simulink model "TosAeb"  (R2021a)
settings: Solver=FixedStepDiscrete  StartTime=0.0  StopTime=inf  FixedStep=0.01  RelTol=1e-3
undefined symbols (not defined in the file or in its folder): ACCModSts, AccSts1, AEB_ObjectMovingOrientation, AEB_ObjectMovingState, CN_BTCActive, CN_CurrentGearR, CN_CurrentGearR_DHT, CN_PTCActive, CN_VDCActive, CN_VSC_EspOff, CN_VSC_FCW_AEB_Error, CN_VSC_FCW_AEB_NoDispay, CN_VSC_FCW_AEB_PerfmDgdtn, CN_VSC_FCW_AEB_TempUnAvlbl, CN_VSC_VehStandstill, CNaccelAvoidMax, CNaccelAvoidMin, CNbrakePedalPress, CNcomfortStrLeftAccelMax, CNcomfortStrLeftAccelMin, CNcomfortStrRightAccelMax, CNcomfortStrRightAccelMin, CNDrvDoorOpen, CNDrvModeEco, CNDrvModeNorm, CNDrvModeOther, CNDrvModeSport, CNDrvSBRreminder, CNNull, CNobjInvalidID, CNPassDoorOpen, CNPassSBRreminder, CNPEBSAccelNegInf, CNPEBSAccelNull, CNPEBSTimeInf, CNPEBSTimeNull, CNSTOPMAX, CNSTOPNULL, CNsubjWidth, CNSwtOFF, CNSwtON, CNTTCMAX, CNTTCNULL, CNVehConfigAEBCycNone, CNVehConfigAEBNone, CNVehConfigAEBPedNone, CNVehConfigFCWNone, CNVehConfigJANone, CollisionSeverity, FALSE, FCWSnvty, JACycObjCalcInfoDefaultParams, JAPedObjCalcInfoDefaultParams, JAVehObjCalcInfoDefaultParams, latCycObjCalcInfoDefaultParams, latPedObjCalcInfoDefaultParams, longObjCalcInfoDefaultParams, ModSts, movingStatus, ObjectType ... (+1112)
libraries: simulink x3031, AEBSFunctionLib x50, Ta_Library x33, AEBSStateMachineLib x23, Mylibrary x12, simulink_extras x7, ObjSelect_lib x3, dspstat3 x2
(part 56 of 102)

== system TosAeb/FcwAebJa_Step_sys/ALG_AEBSystem/ALG_AEBController/AEB_JaObjCalcApp/JaCycTgtInfoCalc/JaCycCollisonAssessInfo/JaCycCollisonAssessInfoCalc/VelAbsTgtCorrCalc/RadicalRelevantCalc ==
  dy : Inport  OutDataTypeStr=single
  dxFrontBumper : Inport  Port=2  OutDataTypeStr=single
  subjYawRate : Inport  Port=3  OutDataTypeStr=single
  Bus Creator : BusCreator  Inputs=2
  Math Function : Math  Operator=hypot  SignedPower=on  SaturateOnIntegerOverflow=on
  Product1 : Product  OutDataTypeStr=single
  Sincos : Trigonometry  Operator=sincos
  Trigonometric Function : Trigonometry  Operator=atan2
  radialVelRel : Outport
  sincosCoef : Outport  Port=2
  wires:
  subjYawRate/1 -> Product1/1
  Math Function/1 -> Product1/2  "dvRadial"
  Product1/1 -> radialVelRel/1  "radialVelRel"
  dy/1 -> Math Function/2, Trigonometric Function/1
  dxFrontBumper/1 -> Trigonometric Function/2, Math Function/1
  Trigonometric Function/1 -> Sincos/1
  Sincos/2 -> Bus Creator/2  "cosCoef"
  Bus Creator/1 -> sincosCoef/1  "sincosCoef"
  Sincos/1 -> Bus Creator/1  "sinCoef"

== system TosAeb/FcwAebJa_Step_sys/ALG_AEBSystem/ALG_AEBController/AEB_JaObjCalcApp/JaCycTgtInfoCalc/JaCycCollisonAssessInfo/JaCycCollisonAssessInfoCalc/VelAbsTgtCorrCalc/RadicalToXYTrans ==
  velRadicalXY : Inport
  sincosCoef : Inport  Port=2
  subjVel : Inport  Port=3  OutDataTypeStr=single
  Add3 : Sum  Inputs=++-  OutDataTypeStr=single
  Add4 : Sum  OutDataTypeStr=single
  Bus Creator4 : BusCreator  Inputs=2
  Bus Selector10 : BusSelector  OutputSignals=velRadicalXY.velRadicalY
  Bus Selector11 : BusSelector  OutputSignals=sincosCoef.sinCoef
  Bus Selector12 : BusSelector  OutputSignals=velRadicalXY.velRadicalX
  Bus Selector13 : BusSelector  OutputSignals=sincosCoef.sinCoef
  Bus Selector14 : BusSelector  OutputSignals=velRadicalXY.velRadicalY
  Bus Selector15 : BusSelector  OutputSignals=sincosCoef.cosCoef
  Bus Selector8 : BusSelector  OutputSignals=velRadicalXY.velRadicalX
  Bus Selector9 : BusSelector  OutputSignals=sincosCoef.cosCoef
  Product{7..10} (4 alike) : Product  OutDataTypeStr=single
  vxAbsTargetCorr : Outport
  vyAbsTargetCorr : Outport  Port=2
  wires:
  Product8/1 -> Add3/3
  Bus Selector13/1 -> Product9/2  "<sinCoef>"
  Add3/1 -> vxAbsTargetCorr/1  "vxAbsTargetCorr"
  Product7/1 -> Add3/2
  Bus Selector14/1 -> Product10/1  "<velRadicalY>"
  Bus Selector11/1 -> Product8/2  "<sinCoef>"
  Bus Selector10/1 -> Product8/1  "<velRadicalY>"
  subjVel/1 -> Add3/1
  Add4/1 -> vyAbsTargetCorr/1  "vyAbsTargetCorr"
  Bus Creator4/1 -> Bus Selector15/1, Bus Selector14/1, Bus Selector13/1, Bus Selector12/1, Bus Selector11/1, Bus Selector10/1, Bus Selector9/1, Bus Selector8/1
  Bus Selector9/1 -> Product7/2  "<cosCoef>"
  Product10/1 -> Add4/2
  Bus Selector15/1 -> Product10/2  "<cosCoef>"
  Bus Selector8/1 -> Product7/1  "<velRadicalX>"
  Product9/1 -> Add4/1
  Bus Selector12/1 -> Product9/1  "<velRadicalX>"
  velRadicalXY/1 -> Bus Creator4/1
  sincosCoef/1 -> Bus Creator4/2

== system TosAeb/FcwAebJa_Step_sys/ALG_AEBSystem/ALG_AEBController/AEB_JaObjCalcApp/JaCycTgtInfoCalc/JaCycCollisonAssessInfo/JaCycCollisonAssessInfoCalc/VelAbsTgtCorrCalc/XYToRadicalTrans ==
  LongLatVelRel : Inport
  radialVelRel : Inport  Port=2  OutDataTypeStr=single
  sincosCoef : Inport  Port=3
  Add : Sum  OutDataTypeStr=single
  Add1 : Sum  OutDataTypeStr=single
  Add2 : Sum  Inputs=+-  OutDataTypeStr=single
  Bus Creator2 : BusCreator  Inputs=2
  Bus Creator3 : BusCreator  Inputs=2
  Bus Selector : BusSelector  OutputSignals=LongLatVelRel.vx
  Bus Selector1 : BusSelector  OutputSignals=sincosCoef.cosCoef
  Bus Selector2 : BusSelector  OutputSignals=LongLatVelRel.vy
  Bus Selector3 : BusSelector  OutputSignals=sincosCoef.sinCoef
  Bus Selector4 : BusSelector  OutputSignals=LongLatVelRel.vy
  Bus Selector5 : BusSelector  OutputSignals=sincosCoef.cosCoef
  Bus Selector6 : BusSelector  OutputSignals=LongLatVelRel.vx
  Bus Selector7 : BusSelector  OutputSignals=sincosCoef.sinCoef
  Product{2,4..6} (4 alike) : Product  OutDataTypeStr=single
  velRadicalXY : Outport
  wires:
  Product6/1 -> Add2/2
  Bus Creator3/1 -> velRadicalXY/1  "velRadicalXY"
  Product5/1 -> Add2/1
  Bus Selector2/1 -> Product4/1  "<vy>"
  Product4/1 -> Add/2
  Product2/1 -> Add/1
  Bus Creator2/1 -> Bus Selector7/1, Bus Selector6/1, Bus Selector5/1, Bus Selector4/1, Bus Selector3/1, Bus Selector2/1, Bus Selector1/1, Bus Selector/1
  Bus Selector5/1 -> Product5/2  "<cosCoef>"
  Add1/1 -> Bus Creator3/2  "velRadicalY"
  Bus Selector6/1 -> Product6/1  "<vx>"
  Add/1 -> Bus Creator3/1  "velRadicalX"
  Bus Selector4/1 -> Product5/1  "<vy>"
  Bus Selector1/1 -> Product2/2  "<cosCoef>"
  radialVelRel/1 -> Add1/2
  Bus Selector3/1 -> Product4/2  "<sinCoef>"
  Bus Selector/1 -> Product2/1  "<vx>"
  Bus Selector7/1 -> Product6/2  "<sinCoef>"
  sincosCoef/1 -> Bus Creator2/2
  LongLatVelRel/1 -> Bus Creator2/1
  Add2/1 -> Add1/1

== system TosAeb/FcwAebJa_Step_sys/ALG_AEBSystem/ALG_AEBController/AEB_JaObjCalcApp/JaCycTgtInfoCalc/JaCycObjCalcInfoCalc/JaCycObjInfoCalc/ObjCrossFlgCal/CollisionProbCalc ==
  tPredTime : Inport  OutDataTypeStr=single
  subjVel : Inport  Port=2  OutDataTypeStr=single
  subjLongAccel : Inport  Port=3  OutDataTypeStr=single
  subjLatAccel : Inport  Port=4  OutDataTypeStr=single
  subjYawRate : Inport  Port=5  OutDataTypeStr=single
  ObjectTypeCameraConfirmed : Inport  Port=6
  ObjectLength : Inport  Port=7  OutDataTypeStr=single
  ObjectWidth : Inport  Port=8  OutDataTypeStr=single
  ObjectMovingOrientation : Inport  Port=9
  dx : Inport  Port=10  OutDataTypeStr=single
  dy : Inport  Port=11  OutDataTypeStr=single
  ObjectID : Inport  Port=12
  vyRelCorr : Inport  Port=13  OutDataTypeStr=single
  vxAbsObj : Inport  Port=14  OutDataTypeStr=single
  ObjectYawAngleCorr : Inport  Port=15  OutDataTypeStr=single
  function : TriggerPort  TriggerType=function-call  VariantControl=Variant  PortDimensions=1
  If : If  IfExpression=u1
  If Action Subsystem : Subsystem  TreatAsAtomicUnit=on
  Merge : Merge
  ObjIsInvalidCalc : Subsystem
  Subsystem : Subsystem
  ObjColliFlg : Outport
  wires:
  tPredTime/1 -> Subsystem/1(tPredTime)
  subjVel/1 -> Subsystem/2(subjVel)
  subjLongAccel/1 -> Subsystem/3(subjLongAccel)
  subjLatAccel/1 -> Subsystem/4(subjLatAccel)
  subjYawRate/1 -> Subsystem/5(subjYawRate)
  ObjectYawAngleCorr/1 -> Subsystem/6(ObjectYawAngleCorr)
  ObjectLength/1 -> Subsystem/8(ObjectLength)
  ObjectWidth/1 -> Subsystem/9(ObjectWidth)
  dx/1 -> Subsystem/11(dx)
  vxAbsObj/1 -> Subsystem/14(vxAbsObj)
  If/1 -> If Action Subsystem/Action
  If Action Subsystem/1(ObjColliFlg) -> Merge/1
  If/2 -> Subsystem/Action
  Merge/1 -> ObjColliFlg/1
  Subsystem/1(ObjColliFlg) -> Merge/2
  dy/1 -> Subsystem/12(dy)
  ObjectID/1 -> ObjIsInvalidCalc/1(ObjectID)
  vyRelCorr/1 -> Subsystem/13(vyRelCorr)
  ObjIsInvalidCalc/1(ObjIsInvalid) -> If/1
  ObjectMovingOrientation/1 -> Subsystem/10(ObjectMovingOrientation)
  ObjectTypeCameraConfirmed/1 -> Subsystem/7(ObjectTypeCameraConfirmed)

== system TosAeb/FcwAebJa_Step_sys/ALG_AEBSystem/ALG_AEBController/AEB_JaObjCalcApp/JaCycTgtInfoCalc/JaCycObjCalcInfoCalc/JaCycObjInfoCalc/SensorTgtInfo2AbsTgtInfoTrans1/ObjFusQualityCal/SensorDxThres ==
  subjVel : Inport
  f : TriggerPort  TriggerType=function-call  VariantControl=(inherit)
      settings: CopyFcn=Stateflow.SLINSF.SimulinkMan.removeBlockPreDeleteFcn  PreDeleteFcn=Stateflow.SLINSF.SimulinkMan.unsafePredeleteFcn
  deltaDxThresCalc : Lookup_n-D  NumberOfTableDimensions=1  BreakpointsForDimension1=P_AEB_JaCycAbs_SensorDeltDxThresX  Table=P_AEB_JaCycAbs_SensorDeltDxThresY  ExtrapMethod=Clip  InternalRulePriority=Speed  RndMeth=Simplest  InputSameDT=on
  deltaDxThres : Outport  OutDataTypeStr=single
  wires:
  subjVel/1 -> deltaDxThresCalc/1
  deltaDxThresCalc/1 -> deltaDxThres/1

== system TosAeb/FcwAebJa_Step_sys/ALG_AEBSystem/ALG_AEBController/AEB_LatObjInfoCalcApp/LatCycObjCalc/LatCycCollisonAssessInfoCalc/LatCycCollisonAssessInfoCalc/DistBrkCondCalc/CycDistBrakeFullCalc ==
  subjLongAccel : Inport  OutDataTypeStr=single
  subjVel : Inport  Port=2
  BrkSys : Subsystem
  Bus Creator : BusCreator  Inputs=6
  Bus Selector : BusSelector  OutputSignals=subjVel,tDelay,subjLongAccelFilt
  Bus Selector1 : BusSelector  OutputSignals=subjVel,Kjerk,subjLongAccelFilt,accelMax
  Bus Selector2 : BusSelector  OutputSignals=tDelay,velDelta,subjVel,subjLongAccelFilt,Kjerk,accelMax
  DistPhase1Calc : Subsystem
  DistPhase2Calc : Subsystem
  DistPhase3Calc : Subsystem
  distBrakeCalc : Subsystem
  distBrake : Outport
  wires:
  DistPhase2Calc/2(egoStopFlg) -> DistPhase3Calc/1(egoStopFlg)
  DistPhase2Calc/3(tJerkWork) -> DistPhase3Calc/2(tJerkWork)
  DistPhase2Calc/4(tPhase2) -> DistPhase3Calc/3(tPhase2)
  DistPhase3Calc/1(distPhase3) -> distBrakeCalc/3(distPhase3)
  DistPhase1Calc/1(distPhase1) -> distBrakeCalc/1(distPhase1)
  DistPhase2Calc/1(distPhase2) -> distBrakeCalc/2(distPhase2)
  distBrakeCalc/1(distBrake) -> distBrake/1
  subjVel/1 -> Bus Creator/1, BrkSys/1(subjVel)  "subjVel"
  BrkSys/3(velDelta) -> Bus Creator/4  "velDelta"
  BrkSys/4(accelMax) -> Bus Creator/5  "accelMax"
  BrkSys/2(Kjerk) -> Bus Creator/3  "Kjerk"
  BrkSys/1(tDelay) -> Bus Creator/2  "tDelay"
  BrkSys/5(subjLongAccelFilt) -> Bus Creator/6  "subjLongAccelFilt"
  Bus Creator/1 -> Bus Selector2/1, Bus Selector1/1, Bus Selector/1
  Bus Selector/3 -> DistPhase1Calc/3(subjLongAccelFilt)  "<subjLongAccelFilt>"
  Bus Selector/2 -> DistPhase1Calc/2(tPhase1)  "<tDelay>"
  Bus Selector/1 -> DistPhase1Calc/1(subjVel)  "<subjVel>"
  Bus Selector1/3 -> DistPhase2Calc/3(subjLongAccelFilt)  "<subjLongAccelFilt>"
  Bus Selector1/2 -> DistPhase2Calc/2(Kjerk)  "<Kjerk>"
  Bus Selector1/1 -> DistPhase2Calc/1(subjVel)  "<subjVel>"
  Bus Selector1/4 -> DistPhase2Calc/4(accelMax)  "<accelMax>"
  Bus Selector2/1 -> DistPhase3Calc/4(tPhase1)  "<tDelay>"
  Bus Selector2/2 -> DistPhase3Calc/5(velDelta)  "<velDelta>"
  Bus Selector2/3 -> DistPhase3Calc/6(subjVel)  "<subjVel>"
  Bus Selector2/4 -> DistPhase3Calc/7(subjLongAccelFilt)  "<subjLongAccelFilt>"
  Bus Selector2/5 -> DistPhase3Calc/8(Kjerk)  "<Kjerk>"
  Bus Selector2/6 -> DistPhase3Calc/9(accelMax)  "<accelMax>"
  subjLongAccel/1 -> BrkSys/2(subjLongAccel)

== system TosAeb/FcwAebJa_Step_sys/ALG_AEBSystem/ALG_AEBController/AEB_LatObjInfoCalcApp/LatCycObjCalc/LatCycCollisonAssessInfoCalc/LatCycCollisonAssessInfoCalc/DistBrkCondCalc/CycDistBrakePartCalc ==
  subjLongAccel : Inport  OutDataTypeStr=single
  subjVel : Inport  Port=2
  BrkSys : Subsystem
  Bus Creator : BusCreator  Inputs=6
  Bus Selector : BusSelector  OutputSignals=subjVel,tDelay,subjLongAccelFilt
  Bus Selector1 : BusSelector  OutputSignals=subjVel,Kjerk,subjLongAccelFilt,accelMax
  Bus Selector2 : BusSelector  OutputSignals=tDelay,velDelta,subjVel,subjLongAccelFilt,Kjerk,accelMax
  DistPhase1Calc : Subsystem
  DistPhase2Calc : Subsystem
  DistPhase3Calc : Subsystem
  distBrakeCalc : Subsystem
  distBrake : Outport
  wires:
  subjLongAccel/1 -> BrkSys/2(subjLongAccel)
  Bus Selector2/6 -> DistPhase3Calc/9(accelMax)  "<accelMax>"
  Bus Selector2/5 -> DistPhase3Calc/8(Kjerk)  "<Kjerk>"
  Bus Selector2/4 -> DistPhase3Calc/7(subjLongAccelFilt)  "<subjLongAccelFilt>"
  Bus Selector2/3 -> DistPhase3Calc/6(subjVel)  "<subjVel>"
  Bus Selector2/2 -> DistPhase3Calc/5(velDelta)  "<velDelta>"
  Bus Selector2/1 -> DistPhase3Calc/4(tPhase1)  "<tDelay>"
  Bus Selector1/4 -> DistPhase2Calc/4(accelMax)  "<accelMax>"
  Bus Selector1/1 -> DistPhase2Calc/1(subjVel)  "<subjVel>"
  Bus Selector1/2 -> DistPhase2Calc/2(Kjerk)  "<Kjerk>"
  Bus Selector1/3 -> DistPhase2Calc/3(subjLongAccelFilt)  "<subjLongAccelFilt>"
  Bus Selector/1 -> DistPhase1Calc/1(subjVel)  "<subjVel>"
  Bus Selector/2 -> DistPhase1Calc/2(tPhase1)  "<tDelay>"
  Bus Selector/3 -> DistPhase1Calc/3(subjLongAccelFilt)  "<subjLongAccelFilt>"
  Bus Creator/1 -> Bus Selector/1, Bus Selector1/1, Bus Selector2/1
  BrkSys/5(subjLongAccelFilt) -> Bus Creator/6  "subjLongAccelFilt"
  BrkSys/1(tDelay) -> Bus Creator/2  "tDelay"
  BrkSys/2(Kjerk) -> Bus Creator/3  "Kjerk"
  BrkSys/4(accelMax) -> Bus Creator/5  "accelMax"
  BrkSys/3(velDelta) -> Bus Creator/4  "velDelta"
  subjVel/1 -> BrkSys/1(subjVel), Bus Creator/1  "subjVel"
  distBrakeCalc/1(distBrake) -> distBrake/1
  DistPhase2Calc/1(distPhase2) -> distBrakeCalc/2(distPhase2)
  DistPhase1Calc/1(distPhase1) -> distBrakeCalc/1(distPhase1)
  DistPhase3Calc/1(distPhase3) -> distBrakeCalc/3(distPhase3)
  DistPhase2Calc/4(tPhase2) -> DistPhase3Calc/3(tPhase2)
  DistPhase2Calc/3(tJerkWork) -> DistPhase3Calc/2(tJerkWork)
  DistPhase2Calc/2(egoStopFlg) -> DistPhase3Calc/1(egoStopFlg)

== system TosAeb/FcwAebJa_Step_sys/ALG_AEBSystem/ALG_AEBController/AEB_LatObjInfoCalcApp/LatCycObjCalc/LatCycCollisonAssessInfoCalc/LatCycCollisonAssessInfoCalc/DistBrkCondCalc/distBraking4FullBrakeCalc1 ==
  subLongAccel : Inport  OutDataTypeStr=single
  subjVel : Inport  Port=2
  Bus Creator : BusCreator  Inputs=2
  Bus Selector : BusSelector  OutputSignals=brkSysAtribute.subjLongAccelCorr,brkSysAtribute.tdelay,subjVel
  Bus Selector1 : BusSelector  OutputSignals=brkSysAtribute.accelMax,brkSysAtribute.subjLongAccelCorr,brkSysAtribute.Kjerk,subjVel
  Bus Selector2 : BusSelector  OutputSignals=brkSysAtribute.subjLongAccelCorr,brkSysAtribute.Kjerk,brkSysAtribute.subjLongAccelCorr,brkSysAtribute.velReduceMax,brkSysAtribute.accelMax
  DistPhase1Calc : Subsystem
  DistPhase2Calc : Subsystem
  brkSysAtributeCalc : Subsystem
  distPhase3Calc : Subsystem
  distanceTriggerBrakingCalc : Subsystem
  distBraking4FullBrake : Outport
  wires:
  Bus Selector2/1 -> distPhase3Calc/3(subjLongAccelCorr)  "<subjLongAccelCorr>"
  Bus Selector2/2 -> distPhase3Calc/4(Kjerk)  "<Kjerk>"
  Bus Selector2/5 -> distPhase3Calc/7(accelMax)  "<accelMax>"
  Bus Selector2/3 -> distPhase3Calc/5(accelWorkPhase1)  "<subjLongAccelCorr>"
  Bus Selector2/4 -> distPhase3Calc/6(velMaxReduce)  "<velReduceMax>"
  [timeWorkPhase1] -> distPhase3Calc/8(tWorkPhase1)
  DistPhase1Calc/2(timeWorkPhase1) -> [timeWorkPhase1]
  DistPhase2Calc/2(Phase2EgoStopFlg) -> distPhase3Calc/1(Phase2EgoStopFlg)
  Bus Selector1/4 -> DistPhase2Calc/4(subjVel)  "<subjVel>"
  DistPhase2Calc/3(tWorkPhase2) -> distPhase3Calc/2(tWorkPhase2)
  brkSysAtributeCalc/1(brkSysAtribute) -> Bus Creator/1  "brkSysAtribute"
  DistPhase1Calc/1(distPhase1) -> distanceTriggerBrakingCalc/1(distPhase1)
  subjVel/1 -> brkSysAtributeCalc/1(subjVel), Bus Creator/2  "subjVel"
  distPhase3Calc/1(distPhase3) -> distanceTriggerBrakingCalc/3(distPhase3)
  subLongAccel/1 -> brkSysAtributeCalc/2(subLongAccel)
  Bus Selector1/2 -> DistPhase2Calc/2(accelWorkPhase1)  "<subjLongAccelCorr>"
  Bus Selector1/1 -> DistPhase2Calc/1(accelMax)  "<accelMax>"
  DistPhase2Calc/1(distPhase2) -> distanceTriggerBrakingCalc/2(distPhase2)
  Bus Selector/1 -> DistPhase1Calc/1(subjlongAccelCorr)  "<subjLongAccelCorr>"
  Bus Selector1/3 -> DistPhase2Calc/3(Kjerk)  "<Kjerk>"
  Bus Selector/3 -> DistPhase1Calc/3(subjVel)  "<subjVel>"
  Bus Selector/2 -> DistPhase1Calc/2(tdelay)  "<tdelay>"
  Bus Creator/1 -> Bus Selector/1, Bus Selector2/1, Bus Selector1/1
  distanceTriggerBrakingCalc/1(DistBraking4FullBrake) -> distBraking4FullBrake/1

== system TosAeb/FcwAebJa_Step_sys/ALG_AEBSystem/ALG_AEBController/AEB_LatObjInfoCalcApp/LatCycObjCalc/LatCycCollisonAssessInfoCalc/LatCycCollisonAssessInfoCalc/DistBrkCondCalc/distBraking4PartBrakeCalc1 ==
  subLongAccel : Inport  OutDataTypeStr=single
  subjVel : Inport  Port=2
  Bus Creator : BusCreator  Inputs=2
  Bus Selector : BusSelector  OutputSignals=brkSysAtribute.subjLongAccelCorr,brkSysAtribute.tdelay,subjVel
  Bus Selector1 : BusSelector  OutputSignals=brkSysAtribute.accelMax,brkSysAtribute.subjLongAccelCorr,brkSysAtribute.Kjerk,subjVel
  Bus Selector2 : BusSelector  OutputSignals=brkSysAtribute.subjLongAccelCorr,brkSysAtribute.Kjerk,brkSysAtribute.subjLongAccelCorr,brkSysAtribute.velReduceMax,brkSysAtribute.accelMax
  DistPhase1Calc : Subsystem
  DistPhase2Calc : Subsystem
  brkSysAtributeCalc : Subsystem
  distPhase3Calc : Subsystem
  distanceTriggerBrakingCalc : Subsystem
  distBraking4PartBrake : Outport
  wires:
  distanceTriggerBrakingCalc/1(distBraking4PartBrake) -> distBraking4PartBrake/1
  Bus Creator/1 -> Bus Selector1/1, Bus Selector2/1, Bus Selector/1
  Bus Selector/2 -> DistPhase1Calc/2(tdelay)  "<tdelay>"
  Bus Selector/3 -> DistPhase1Calc/3(subjVel)  "<subjVel>"
  Bus Selector1/3 -> DistPhase2Calc/3(Kjerk)  "<Kjerk>"
  Bus Selector/1 -> DistPhase1Calc/1(subjlongAccelCorr)  "<subjLongAccelCorr>"
  DistPhase2Calc/1(distPhase2) -> distanceTriggerBrakingCalc/2(distPhase2)
  Bus Selector1/1 -> DistPhase2Calc/1(accelMax)  "<accelMax>"
  Bus Selector1/2 -> DistPhase2Calc/2(accelWorkPhase1)  "<subjLongAccelCorr>"
  subLongAccel/1 -> brkSysAtributeCalc/2(subLongAccel)
  distPhase3Calc/1(distPhase3) -> distanceTriggerBrakingCalc/3(distPhase3)
  subjVel/1 -> Bus Creator/2, brkSysAtributeCalc/1(subjVel)  "subjVel"
  DistPhase1Calc/1(distPhase1) -> distanceTriggerBrakingCalc/1(distPhase1)
  brkSysAtributeCalc/1(brkSysAtribute) -> Bus Creator/1  "brkSysAtribute"
  DistPhase2Calc/3(tWorkPhase2) -> distPhase3Calc/2(tWorkPhase2)
  Bus Selector1/4 -> DistPhase2Calc/4(subjVel)  "<subjVel>"
  DistPhase2Calc/2(Phase2EgoStopFlg) -> distPhase3Calc/1(Phase2EgoStopFlg)
  DistPhase1Calc/2(timeWorkPhase1) -> [timeWorkPhase1]
  [timeWorkPhase1] -> distPhase3Calc/8(tWorkPhase1)
  Bus Selector2/4 -> distPhase3Calc/6(velMaxReduce)  "<velReduceMax>"
  Bus Selector2/3 -> distPhase3Calc/5(accelWorkPhase1)  "<subjLongAccelCorr>"
  Bus Selector2/5 -> distPhase3Calc/7(accelMax)  "<accelMax>"
  Bus Selector2/2 -> distPhase3Calc/4(Kjerk)  "<Kjerk>"
  Bus Selector2/1 -> distPhase3Calc/3(subjLongAccelCorr)  "<subjLongAccelCorr>"

== system TosAeb/FcwAebJa_Step_sys/ALG_AEBSystem/ALG_AEBController/AEB_LatObjInfoCalcApp/LatCycObjCalc/LatCycCollisonAssessInfoCalc/LatCycCollisonAssessInfoCalc/DistBrkCondCalc/distSecurCalc ==
  subVel : Inport
  Compare To Constant : simulink/Logic and Bit Operations/Compare To Constant  relop=>  const=P_AEB_LatCycCA_DxSecureSubjVelThres  OutDataTypeStr=boolean
  If : If  IfExpression=u1
  If Action Subsystem : Subsystem  TreatAsAtomicUnit=on
  If Action Subsystem1 : Subsystem  TreatAsAtomicUnit=on
  Merge : Merge
  distSecure : Outport
  wires:
  Merge/1 -> distSecure/1
  If Action Subsystem/1(distSecure) -> Merge/2
  If Action Subsystem1/1(distSecure) -> Merge/1
  If/2 -> If Action Subsystem/Action
  If/1 -> If Action Subsystem1/Action
  Compare To Constant/1 -> If/1
  subVel/1 -> Compare To Constant/1, If Action Subsystem/1(subjVel)

== system TosAeb/FcwAebJa_Step_sys/ALG_AEBSystem/ALG_AEBController/AEB_LatObjInfoCalcApp/LatCycObjCalc/LatCycObjCalc/TgtPreSelectCalc/TgtPreSelect/Object Cond  ==
  ObjectType : Inport
  ObjectTypeCameraConfirmed : Inport  Port=2
  ObjectIsObstacleProb : Inport  Port=3
  Compare To Constant6 : simulink/Logic and Bit Operations/Compare To Constant  relop===  const=TRUE  OutDataTypeStr=boolean
  Compare To Constant7 : simulink/Logic and Bit Operations/Compare To Constant  relop===  const=ObjectType.BICYCLE  OutDataTypeStr=boolean
  Compare To Constant8 : simulink/Logic and Bit Operations/Compare To Constant  relop===  const=ObjectType.BICYCLE  OutDataTypeStr=boolean
  Compare To Constant9 : simulink/Logic and Bit Operations/Compare To Constant  relop=>  const=P_AEB_LatCycCA_PreSelectObjectIsObstacleProbThres  OutDataTypeStr=boolean
  Logical Operator : Logic  AllPortsSameDT=off  OutDataTypeStr=boolean
  Switch : Switch  Criteria=u2 ~= 0
  Out1 : Outport
  wires:
  Switch/1 -> Out1/1
  Logical Operator/1 -> Switch/3
  Compare To Constant9/1 -> Logical Operator/2
  ObjectType/1 -> Compare To Constant7/1, Compare To Constant8/1
  Compare To Constant7/1 -> Logical Operator/1
  Compare To Constant6/1 -> Switch/2
  ObjectTypeCameraConfirmed/1 -> Compare To Constant6/1
  Compare To Constant8/1 -> Switch/1
  ObjectIsObstacleProb/1 -> Compare To Constant9/1

== system TosAeb/FcwAebJa_Step_sys/ALG_AEBSystem/ALG_AEBController/AEB_LatObjInfoCalcApp/LatCycObjCalc/LatCycObjCalc/latCycObjInfoCalc/ObjColiisionRiskProbCalc/CollisionRiskProbCalc ==
  tPredTime : Inport
  ObjectTypeCameraConfirmed : Inport  Port=2
  ObjectLength : Inport  Port=3
  ObjectWidth : Inport  Port=4
  ObjectYawAngle : Inport  Port=5
  ObjectMovingOrientation : Inport  Port=6
  vx : Inport  Port=7
  vy : Inport  Port=8
  dy : Inport  Port=9
  dx : Inport  Port=10
  subjVel : Inport  Port=11
  subjYawRate : Inport  Port=12
  dxFrontBumper : Inport  Port=13
  vxAbsObj : Inport  Port=14
  function : TriggerPort  TriggerType=function-call  VariantControl=Variant  PortDimensions=1
  Bus Creator : BusCreator  Inputs=14
  Bus Selector : BusSelector  OutputSignals=tPredTime,subjVel
  Bus Selector1 : BusSelector  OutputSignals=vx,vy,dy,dxFrontBumper,subjYawRate,subjVel
  Bus Selector2 : BusSelector  OutputSignals=tPredTime,ObjectTypeCameraConfirmed,ObjectWidth,ObjectYawAngle,ObjectMovingOrientation,ObjectLength,dx,dy,vxAbsObj,vy
  CollisionRiskProbCalc : Subsystem
  Display : Display
  Display1 : Display
  Display2 : Display
  EgoReginCalc : Subsystem
  EgoReginCorrCalc : Subsystem
  MATLAB Function : MATLAB Function  Commented=on  TreatAsAtomicUnit=on
  ObjAbsInfoCalc : Subsystem
  ObjReginCorrCalc : Subsystem
  Terminator1 : Terminator
  Terminator2 : Terminator
  collisionRiskProb : Outport
  dyCollisionRiskProb : Outport  Port=2
  dxCollisionRiskProb : Outport  Port=3
  wires:
  CollisionRiskProbCalc/5(dxCollisionRiskProb) -> Display1/1, [dxCollisionRiskProb], dxCollisionRiskProb/1
  CollisionRiskProbCalc/4(dyCollisionRiskProb) -> Display/1, [dyCollisionRiskProb], dyCollisionRiskProb/1
  ObjReginCorrCalc/4(dyReginObjLLCorr) -> CollisionRiskProbCalc/8(dyReginObjLLCorr), [dyReginObjLLCorr]
  ObjReginCorrCalc/3(dxReginObjLLCorr) -> CollisionRiskProbCalc/7(dxReginObjLLCorr), [dxReginObjLLCorr]
  ObjReginCorrCalc/2(dyReginObjRUCorr) -> CollisionRiskProbCalc/6(dyReginObjRUCorr), [dyReginObjRUCorr]
  ObjReginCorrCalc/1(dxReginObjRUCorr) -> CollisionRiskProbCalc/5(dxReginObjRUCorr), [dxReginObjRUCorr]
  EgoReginCalc/4(dyReginEgoLL) -> EgoReginCorrCalc/4(dyReginEgoLL)
  EgoReginCalc/3(dxReginEgoLL) -> EgoReginCorrCalc/3(dxReginEgoLL)
  EgoReginCalc/2(dyReginEgoRU) -> EgoReginCorrCalc/2(dyReginEgoRU)
  EgoReginCalc/1(dxReginEgoRU) -> EgoReginCorrCalc/1(dxReginEgoRU)
  Bus Selector1/6 -> ObjAbsInfoCalc/6(subjVel)  "<subjVel>"
  Bus Selector1/5 -> ObjAbsInfoCalc/5(subjYawRate)  "<subjYawRate>"
  Bus Selector1/4 -> ObjAbsInfoCalc/4(dxFrontBumper)  "<dxFrontBumper>"
  Bus Selector1/3 -> ObjAbsInfoCalc/3(dy)  "<dy>"
  Bus Selector1/2 -> ObjAbsInfoCalc/2(vy)  "<vy>"
  Bus Selector1/1 -> ObjAbsInfoCalc/1(vx)  "<vx>"
  subjYawRate/1 -> Bus Creator/13  "subjYawRate"
  subjVel/1 -> Bus Creator/12  "subjVel"
  dxFrontBumper/1 -> Bus Creator/11  "dxFrontBumper"
  dy/1 -> [dy], Bus Creator/10  "dy"
  dx/1 -> [dx], Bus Creator/9  "dx"
  Bus Selector/2 -> EgoReginCalc/2(subjVel)  "<subjVel>"
  Bus Selector/1 -> EgoReginCalc/1(tPredTime)  "<tPredTime>"
  Bus Creator/1 -> Bus Selector2/1, Bus Selector1/1, Bus Selector/1
  vy/1 -> Bus Creator/8  "vy"
  vx/1 -> Bus Creator/7  "vx"
  ObjectYawAngle/1 -> Bus Creator/5  "ObjectYawAngle"
  ObjectWidth/1 -> Bus Creator/4  "ObjectWidth"
  ObjectLength/1 -> Bus Creator/3  "ObjectLength"
  ObjectTypeCameraConfirmed/1 -> Bus Creator/2  "ObjectTypeCameraConfirmed"
  tPredTime/1 -> Bus Creator/1  "tPredTime"
  ObjAbsInfoCalc/1(velAbsObjCorr) -> ObjReginCorrCalc/1(velAbsObjCorr)
  CollisionRiskProbCalc/3(collisionRiskProb) -> Display2/1, [collisionRiskProb], collisionRiskProb/1
  CollisionRiskProbCalc/2(dyPreCollison) -> Terminator2/1
  CollisionRiskProbCalc/1(dxPreCollison) -> Terminator1/1
  EgoReginCorrCalc/2(dyReginEgoRUCorr) -> [dyReginEgoRUCorr], CollisionRiskProbCalc/2(dyReginEgoRUCorr)
  EgoReginCorrCalc/3(dyReginEgoLLCorr) -> [dyReginEgoLLCorr], CollisionRiskProbCalc/3(dyReginEgoLLCorr)
  EgoReginCorrCalc/1(dxReginEgoRUCorr) -> [dxReginEgoRUCorr], CollisionRiskProbCalc/1(dxReginEgoRUCorr)
  EgoReginCorrCalc/4(dxReginEgoLLCorr) -> [dxReginEgoLLCorr], CollisionRiskProbCalc/4(dxReginEgoLLCorr)
  Bus Selector2/1 -> ObjReginCorrCalc/2(tPredTime)  "<tPredTime>"
  Bus Selector2/3 -> ObjReginCorrCalc/4(ObjectWidth)  "<ObjectWidth>"
  Bus Selector2/4 -> ObjReginCorrCalc/5(ObjectYawAngle)  "<ObjectYawAngle>"
  Bus Selector2/6 -> ObjReginCorrCalc/7(ObjectLength)  "<ObjectLength>"
  Bus Selector2/7 -> ObjReginCorrCalc/8(dx)  "<dx>"
  Bus Selector2/8 -> ObjReginCorrCalc/9(dy)  "<dy>"
  Bus Selector2/9 -> ObjReginCorrCalc/10(vxAbsObj)  "<vxAbsObj>"
  Bus Selector2/10 -> ObjReginCorrCalc/11(vy)  "<vy>"
  vxAbsObj/1 -> Bus Creator/14  "vxAbsObj"
  [dyReginObjRUCorr] -> MATLAB Function/6(dyReginObjRUCorr)
  [dyCollisionRiskProb] -> MATLAB Function/12(dyCollisionRiskProb)
  [collisionRiskProb] -> MATLAB Function/11(collisionRiskProb)
  [dxCollisionRiskProb] -> MATLAB Function/13(dxCollisionRiskProb)
  [dxReginObjLLCorr] -> MATLAB Function/7(dxReginObjLLCorr)
  [dyReginObjLLCorr] -> MATLAB Function/8(dyReginObjLLCorr)
  [dxReginEgoRUCorr] -> MATLAB Function/1(dxReginEgoRUCorr)
  [dxReginObjRUCorr] -> MATLAB Function/5(dxReginObjRUCorr)
  [dxReginEgoLLCorr] -> MATLAB Function/4(dxReginEgoLLCorr)
  [dyReginEgoLLCorr] -> MATLAB Function/3(dyReginEgoLLCorr)
  [dyReginEgoRUCorr] -> MATLAB Function/2(dyReginEgoRUCorr)
  [dx] -> MATLAB Function/9(dx)
  [dy] -> MATLAB Function/10(dy)
  ObjectMovingOrientation/1 -> Bus Creator/6
  Bus Selector2/5 -> ObjReginCorrCalc/6(ObjectMovingOrientation)  "<ObjectMovingOrientation>"
  Bus Selector2/2 -> ObjReginCorrCalc/3(ObjectTypeCameraConfirmed)  "<ObjectTypeCameraConfirmed>"

== system TosAeb/FcwAebJa_Step_sys/ALG_AEBSystem/ALG_AEBController/AEB_LatObjInfoCalcApp/LatCycObjCalc/LatCycObjCalc/latCycObjInfoCalc/ObjCrossFlgCal/CycPreCollisionCalcFunc ==
  tPredTime : Inport  OutDataTypeStr=single
  subjVel : Inport  Port=2
  subjLongAccel : Inport  Port=3
  subjLatAccel : Inport  Port=4
  subjYawRate : Inport  Port=5
  ObjectTypeCameraConfirmed : Inport  Port=6
  ObjectLength : Inport  Port=7
  ObjectWidth : Inport  Port=8
  ObjectYawAngle : Inport  Port=9
  ObjectMovingOrientation : Inport  Port=10
  dx : Inport  Port=11
  vy : Inport  Port=12
  ay : Inport  Port=13
  dy : Inport  Port=14
  vxAbsObj : Inport  Port=15
  axAbsObj : Inport  Port=16
  function : TriggerPort  TriggerType=function-call  VariantControl=Variant  PortDimensions=1
  ObjBoundingBox : Subsystem
  ObjLenthWidthCalc : Subsystem
  ObjPreReferPointCalc : Subsystem
  PreCrossFlgCalcFlow : Stateflow chart  TreatAsAtomicUnit=on
  PreRefPointEgoCalc : Subsystem
  SubjBoundingBox : Subsystem
  sincosCoefCalc : Subsystem
  sincosCoefCalc1 : Subsystem
  pedPreCollisionFlg : Outport
  wires:
  [subjLatAccel] -> ObjPreReferPointCalc/6(subjLatAccel)
  [tPredTime] -> ObjPreReferPointCalc/3(tPredTime)
  subjYawRate/1 -> PreRefPointEgoCalc/5(subjYawRate)
  subjLatAccel/1 -> PreRefPointEgoCalc/4(subjLatAccel), [subjLatAccel]
  subjVel/1 -> PreRefPointEgoCalc/1(subjVel)
  subjLongAccel/1 -> PreRefPointEgoCalc/3(subjLongAccel)
  tPredTime/1 -> PreRefPointEgoCalc/2(tPredTime), [tPredTime]
  dy/1 -> ObjPreReferPointCalc/8(dy)
  ay/1 -> ObjPreReferPointCalc/7(ay)
  vy/1 -> ObjPreReferPointCalc/5(vy)
  axAbsObj/1 -> ObjPreReferPointCalc/4(axAbsObj)
  vxAbsObj/1 -> ObjPreReferPointCalc/2(vxAbsObj)
  dx/1 -> ObjPreReferPointCalc/1(dx)
  ObjectWidth/1 -> ObjLenthWidthCalc/3(ObjectWidth)
  ObjectLength/1 -> ObjLenthWidthCalc/2(ObjectLength)
  PreCrossFlgCalcFlow/1(pedPreCollisionFlg) -> pedPreCollisionFlg/1
  ObjBoundingBox/2(objDyPre) -> PreCrossFlgCalcFlow/4(objDyPre)
  ObjBoundingBox/1(objDxPre) -> PreCrossFlgCalcFlow/3(objDxPre)
  SubjBoundingBox/2(subjDyPre) -> PreCrossFlgCalcFlow/2(subjDyPre)
  SubjBoundingBox/1(subjDxPre) -> PreCrossFlgCalcFlow/1(subjDxPre)
  sincosCoefCalc1/1(sincosCoef) -> ObjBoundingBox/5(sincosCoef)
  ObjPreReferPointCalc/2(dyPreRefPointObj) -> ObjBoundingBox/4(dyPreRefPointObj)
  ObjPreReferPointCalc/1(dxPreRefPointObj) -> ObjBoundingBox/3(dxPreRefPointObj)
  ObjLenthWidthCalc/1(ObjLengthCorr) -> ObjBoundingBox/1(ObjLengthCorr)
  ObjLenthWidthCalc/2(ObjWidthCorr) -> ObjBoundingBox/2(ObjWidthCorr)
  ObjPreReferPointCalc/3(YawAnglePreObj) -> sincosCoefCalc1/1(angle)
  PreRefPointEgoCalc/1(" dxPreRefPoint") -> SubjBoundingBox/1(dxPreRefPoint)
  sincosCoefCalc/1(sincosCoef) -> SubjBoundingBox/3(sincosCoef)
  PreRefPointEgoCalc/2(" dyPreRefPoint") -> SubjBoundingBox/2(dyPreRefPoint)
  PreRefPointEgoCalc/3(YawAnglePre) -> sincosCoefCalc/1(angle)
  ObjectMovingOrientation/1 -> ObjLenthWidthCalc/5(ObjectMovingOrientation)
  ObjectTypeCameraConfirmed/1 -> ObjLenthWidthCalc/1(ObjectTypeCameraConfirmed)
  ObjectYawAngle/1 -> ObjPreReferPointCalc/9(ObjectYawAngle), ObjLenthWidthCalc/4(ObjectYawAngle)

== system TosAeb/FcwAebJa_Step_sys/ALG_AEBSystem/ALG_AEBController/AEB_LatObjInfoCalcApp/LatCycObjCalc/LatCycObjCalc/latCycObjInfoCalc/SensorTgtInfo2AbsTgtInfoTrans/ObjFusQualityCal/SensorVyThres ==
  subjVel : Inport
  f : TriggerPort  TriggerType=function-call  VariantControl=(inherit)
      settings: CopyFcn=Stateflow.SLINSF.SimulinkMan.removeBlockPreDeleteFcn  PreDeleteFcn=Stateflow.SLINSF.SimulinkMan.unsafePredeleteFcn
  deltaDxThresCalc : Lookup_n-D  NumberOfTableDimensions=1  BreakpointsForDimension1=P_AEB_LatCycObjAbs_SensorDeltDxThresX  Table=P_AEB_LatCycObjAbs_SensorDeltDxThresY  ExtrapMethod=Clip  InternalRulePriority=Speed  RndMeth=Simplest  InputSameDT=on
  deltaDyThresCalc : Lookup_n-D  NumberOfTableDimensions=1  BreakpointsForDimension1=P_AEB_LatCycObjAbs_SensorDeltDyThresX  Table=P_AEB_LatCycObjAbs_SensorDeltDyThresY  ExtrapMethod=Clip  InternalRulePriority=Speed  RndMeth=Simplest  InputSameDT=on
  deltaVyThresCalc : Lookup_n-D  NumberOfTableDimensions=1  BreakpointsForDimension1=P_AEB_LatCycObjAbs_SensorDeltVyThresX  Table=P_AEB_LatCycObjAbs_SensorDeltVyThresY  ExtrapMethod=Clip  InternalRulePriority=Speed  RndMeth=Simplest  InputSameDT=on
  deltaDxThres : Outport  OutDataTypeStr=single
  deltaDyThres : Outport  Port=2  OutDataTypeStr=single
  deltaVyThres : Outport  Port=3  OutDataTypeStr=single
  wires:
  subjVel/1 -> deltaDxThresCalc/1, deltaDyThresCalc/1, deltaVyThresCalc/1
  deltaVyThresCalc/1 -> deltaVyThres/1
  deltaDxThresCalc/1 -> deltaDxThres/1
  deltaDyThresCalc/1 -> deltaDyThres/1

== system TosAeb/FcwAebJa_Step_sys/ALG_AEBSystem/ALG_AEBController/AEB_LatObjInfoCalcApp/LatPedObjCalc/LatPedCollisonAssessInfo/LatPedCollisonAssessInfo/LatPedCollisionSeverityCalc/longDistCond4CollisionSeverityHighCalc ==
  distSecure : Inport
  distBraking : Inport  Port=2  OutDataTypeStr=single
  dxFrontBumper : Inport  Port=3  OutDataTypeStr=single
  vx : Inport  Port=4
  Add : Sum  Inputs=++-  OutDataTypeStr=single
  P_LatPedCA_tSeverityHighTimeOffset : Constant  Value=P_AEB_LatPedCA_tSeverityHighTimeOffset
  Product : Product
  Relational Operator : RelationalOperator  Operator=<=  OutDataTypeStr=boolean  RndMeth=Simplest
  longDistCond4CollisionSeverityHigh : Outport
  wires:
  Add/1 -> Relational Operator/2
  vx/1 -> Product/1
  dxFrontBumper/1 -> Relational Operator/1
  Relational Operator/1 -> longDistCond4CollisionSeverityHigh/1
  distSecure/1 -> Add/1
  distBraking/1 -> Add/2
  P_LatPedCA_tSeverityHighTimeOffset/1 -> Product/2
  Product/1 -> Add/3

== system TosAeb/FcwAebJa_Step_sys/ALG_AEBSystem/ALG_AEBController/AEB_LatObjInfoCalcApp/LatPedObjCalc/LatPedCollisonAssessInfo/LatPedCollisonAssessInfo/LatPedCollisionSeverityCalc/longDistCond4CollisionSeverityLowCalc ==
  dxFrontBumper : Inport  OutDataTypeStr=single
  distSecure : Inport  Port=2
  distBraking : Inport  Port=3  OutDataTypeStr=single
  subjVel : Inport  Port=4
  Add : Sum  Inputs=+++  OutDataTypeStr=single
  P_LatPedCA_SeverityLowTimeOffset : Constant  Value=P_AEB_LatPedCA_SeverityLowTimeOffset
  Product : Product
  Relational Operator : RelationalOperator  Operator=<=  OutDataTypeStr=boolean  RndMeth=Simplest
  longDistCond4CollisionSeverityLow : Outport
  wires:
  distSecure/1 -> Add/1
  distBraking/1 -> Add/2
  Add/1 -> Relational Operator/2
  dxFrontBumper/1 -> Relational Operator/1
  Relational Operator/1 -> longDistCond4CollisionSeverityLow/1
  subjVel/1 -> Product/1
  Product/1 -> Add/3
  P_LatPedCA_SeverityLowTimeOffset/1 -> Product/2

== system TosAeb/FcwAebJa_Step_sys/ALG_AEBSystem/ALG_AEBController/AEB_LatObjInfoCalcApp/LatPedObjCalc/LatPedCollisonAssessInfo/LatPedCollisonAssessInfo/LatPedCollisionSeverityCalc/longDistCond4CollisionSeverityMediumCalc ==
  FcwHMISetting : Inport
  subjVel : Inport  Port=2
  dxFrontBumper : Inport  Port=3  OutDataTypeStr=single
  Compare To Constant : simulink/Logic and Bit Operations/Compare To Constant  relop===  const=FCWSnvty.HMISettingEarly  OutDataTypeStr=boolean
  Compare To Constant1 : simulink/Logic and Bit Operations/Compare To Constant  relop===  const=FCWSnvty.HMISettingMiddle  OutDataTypeStr=boolean
  Logical Operator : Logic  Operator=OR  AllPortsSameDT=off  OutDataTypeStr=boolean
  P_LatPedCA_SeverityMediumMaxTime : Constant  Value=P_AEB_LatPedCA_SeverityMediumMaxTime  OutDataTypeStr=single
  P_LatPedCA_SeverityMediumMaxTimeHmiLate : Constant  Value=P_AEB_LatPedCA_SeverityMediumMaxTimeHmiLate  OutDataTypeStr=single
  Product : Product  OutDataTypeStr=single
  Relational Operator : RelationalOperator  Operator=<=  OutDataTypeStr=boolean  RndMeth=Simplest
  Switch : Switch  Criteria=u2 ~= 0  OutDataTypeStr=single
  longDistCond4CollisionSeverityMedium : Outport
  wires:
  FcwHMISetting/1 -> Compare To Constant1/1, Compare To Constant/1
  Compare To Constant/1 -> Logical Operator/1
  Compare To Constant1/1 -> Logical Operator/2
  Logical Operator/1 -> Switch/2
  P_LatPedCA_SeverityMediumMaxTime/1 -> Switch/1
  P_LatPedCA_SeverityMediumMaxTimeHmiLate/1 -> Switch/3
  Switch/1 -> Product/1
  subjVel/1 -> Product/2
  Product/1 -> Relational Operator/2
  dxFrontBumper/1 -> Relational Operator/1
  Relational Operator/1 -> longDistCond4CollisionSeverityMedium/1

== system TosAeb/FcwAebJa_Step_sys/ALG_AEBSystem/ALG_AEBController/AEB_LatObjInfoCalcApp/LatPedObjCalc/LatPedCollisonAssessInfo/LatPedCollisonAssessInfo/LatPedLongDistCondCalc/DistSecureCalc ==
  subjVel : Inport
  Compare To Constant : simulink/Logic and Bit Operations/Compare To Constant  relop=>  const=P_AEB_LatPedCA_DxSecureSubjVelThres  OutDataTypeStr=boolean
  If : If  IfExpression=u1
  If Action Subsystem : Subsystem  TreatAsAtomicUnit=on
  If Action Subsystem1 : Subsystem  TreatAsAtomicUnit=on
  Merge : Merge
  distSecure : Outport
  wires:
  Merge/1 -> distSecure/1  "distSecure"
  If Action Subsystem/1(distSecure) -> Merge/2
  If Action Subsystem1/1(distSecure) -> Merge/1
  subjVel/1 -> Compare To Constant/1, If Action Subsystem/1(subjVel)
  If/2 -> If Action Subsystem/Action
  Compare To Constant/1 -> If/1
  If/1 -> If Action Subsystem1/Action

== system TosAeb/FcwAebJa_Step_sys/ALG_AEBSystem/ALG_AEBController/AEB_LatObjInfoCalcApp/LatPedObjCalc/LatPedCollisonAssessInfo/LatPedCollisonAssessInfo/LatPedLongDistCondCalc/PedDistBrakeFullCalc ==
  subjLongAccel : Inport  OutDataTypeStr=single
  subjVel : Inport  Port=2
  BrkSys : Subsystem
  Bus Creator : BusCreator  Inputs=6
  Bus Selector : BusSelector  OutputSignals=subjVel,tDelay,subjLongAccelFilt
  Bus Selector1 : BusSelector  OutputSignals=subjVel,Kjerk,subjLongAccelFilt,accelMax
  Bus Selector2 : BusSelector  OutputSignals=tDelay,velDelta,subjVel,subjLongAccelFilt,Kjerk,accelMax
  DistPhase1Calc : Subsystem
  DistPhase2Calc : Subsystem
  DistPhase3Calc : Subsystem
  distBrakeCalc : Subsystem
  distBrake : Outport
  wires:
  DistPhase2Calc/2(egoStopFlg) -> DistPhase3Calc/1(egoStopFlg)
  DistPhase2Calc/3(tJerkWork) -> DistPhase3Calc/2(tJerkWork)
  DistPhase2Calc/4(tPhase2) -> DistPhase3Calc/3(tPhase2)
  DistPhase3Calc/1(distPhase3) -> distBrakeCalc/3(distPhase3)
  DistPhase1Calc/1(distPhase1) -> distBrakeCalc/1(distPhase1)
  DistPhase2Calc/1(distPhase2) -> distBrakeCalc/2(distPhase2)
  distBrakeCalc/1(distBrake) -> distBrake/1
  subjVel/1 -> Bus Creator/1, BrkSys/1(subjVel)  "subjVel"
  BrkSys/3(velDelta) -> Bus Creator/4  "velDelta"
  BrkSys/4(accelMax) -> Bus Creator/5  "accelMax"
  BrkSys/2(Kjerk) -> Bus Creator/3  "Kjerk"
  BrkSys/1(tDelay) -> Bus Creator/2  "tDelay"
  BrkSys/5(subjLongAccelFilt) -> Bus Creator/6  "subjLongAccelFilt"
  Bus Creator/1 -> Bus Selector2/1, Bus Selector1/1, Bus Selector/1
  Bus Selector/3 -> DistPhase1Calc/3(subjLongAccelFilt)  "<subjLongAccelFilt>"
  Bus Selector/2 -> DistPhase1Calc/2(tPhase1)  "<tDelay>"
  Bus Selector/1 -> DistPhase1Calc/1(subjVel)  "<subjVel>"
  Bus Selector1/3 -> DistPhase2Calc/3(subjLongAccelFilt)  "<subjLongAccelFilt>"
  Bus Selector1/2 -> DistPhase2Calc/2(Kjerk)  "<Kjerk>"
  Bus Selector1/1 -> DistPhase2Calc/1(subjVel)  "<subjVel>"
  Bus Selector1/4 -> DistPhase2Calc/4(accelMax)  "<accelMax>"
  Bus Selector2/1 -> DistPhase3Calc/4(tPhase1)  "<tDelay>"
  Bus Selector2/2 -> DistPhase3Calc/5(velDelta)  "<velDelta>"
  Bus Selector2/3 -> DistPhase3Calc/6(subjVel)  "<subjVel>"
  Bus Selector2/4 -> DistPhase3Calc/7(subjLongAccelFilt)  "<subjLongAccelFilt>"
  Bus Selector2/5 -> DistPhase3Calc/8(Kjerk)  "<Kjerk>"
  Bus Selector2/6 -> DistPhase3Calc/9(accelMax)  "<accelMax>"
  subjLongAccel/1 -> BrkSys/2(subjLongAccel)

== system TosAeb/FcwAebJa_Step_sys/ALG_AEBSystem/ALG_AEBController/AEB_LatObjInfoCalcApp/LatPedObjCalc/LatPedObjCalc/ObjPreSelectCondCalc/TgtPreSelect/ObjectTypeCond ==
  ObjectType : Inport
  ObjectTypeCameraConfirmed : Inport  Port=2
  ObjectIsObstacleProb : Inport  Port=3
  Compare To Constant6 : simulink/Logic and Bit Operations/Compare To Constant  relop===  const=TRUE  OutDataTypeStr=boolean
  Compare To Constant7 : simulink/Logic and Bit Operations/Compare To Constant  relop===  const=ObjectType.PEDESTRIAN  OutDataTypeStr=boolean
  Compare To Constant8 : simulink/Logic and Bit Operations/Compare To Constant  relop===  const=ObjectType.PEDESTRIAN  OutDataTypeStr=boolean
  Compare To Constant9 : simulink/Logic and Bit Operations/Compare To Constant  relop=>  const=P_AEB_LatPedCA_PreSelectObjectIsObstacleProbThres  OutDataTypeStr=boolean
  Logical Operator : Logic  AllPortsSameDT=off  OutDataTypeStr=boolean
  Switch : Switch  Criteria=u2 ~= 0
  Out1 : Outport
  wires:
  Logical Operator/1 -> Switch/3
  Compare To Constant9/1 -> Logical Operator/2
  ObjectTypeCameraConfirmed/1 -> Compare To Constant6/1
  Compare To Constant6/1 -> Switch/2
  ObjectType/1 -> Compare To Constant8/1, Compare To Constant7/1
  Compare To Constant8/1 -> Logical Operator/1
  Compare To Constant7/1 -> Switch/1
  ObjectIsObstacleProb/1 -> Compare To Constant9/1
  Switch/1 -> Out1/1

== system TosAeb/FcwAebJa_Step_sys/ALG_AEBSystem/ALG_AEBController/AEB_LatObjInfoCalcApp/LatPedObjCalc/LatPedObjCalc/latPedObjInfoCalc/ObjColiisionRiskProbCalc/CollisionRiskProbCalc ==
  tPredTime : Inport  OutDataTypeStr=single
  ObjectTypeCameraConfirmed : Inport  Port=2  OutDataTypeStr=boolean
  ObjectLength : Inport  Port=3
  ObjectWidth : Inport  Port=4
  ObjectYawAngle : Inport  Port=5
  vx : Inport  Port=6
  vy : Inport  Port=7
  dy : Inport  Port=8
  dx : Inport  Port=9
  ObjectMovingOrientation : Inport  Port=10
  subjVel : Inport  Port=11
  subjYawRate : Inport  Port=12
  dxFrontBumper : Inport  Port=13
  function : TriggerPort  TriggerType=function-call  VariantControl=Variant  PortDimensions=1
  Bus Creator : BusCreator  Inputs=13
  Bus Selector : BusSelector  OutputSignals=tPredTime,subjVel
  Bus Selector1 : BusSelector  OutputSignals=vx,vy,dy,dxFrontBumper,subjYawRate,subjVel
  Bus Selector2 : BusSelector  OutputSignals=tPredTime,ObjectTypeCameraConfirmed,ObjectLength,ObjectWidth,ObjectYawAngle,ObjectMovingOrientation,dx,dy
  CollisionRiskProbCalc : Subsystem
  EgoReginCalc : Subsystem
  EgoReginCorrCalc : Subsystem
  MATLAB Function : MATLAB Function  Commented=on  TreatAsAtomicUnit=on
  ObjAbsInfoCalc : Subsystem
  ObjReginCorrCalc : Subsystem
  Terminator1 : Terminator
  Terminator2 : Terminator
  collisionRiskProb : Outport
  dyCollisionRiskProb : Outport  Port=2
  dxCollisionRiskProb : Outport  Port=3
  wires:
  EgoReginCorrCalc/4(dxReginEgoLLCorr) -> [dxReginEgoLLCorr], CollisionRiskProbCalc/4(dxReginEgoLLCorr)
  EgoReginCorrCalc/1(dxReginEgoRUCorr) -> [dxReginEgoRUCorr], CollisionRiskProbCalc/1(dxReginEgoRUCorr)
  EgoReginCorrCalc/3(dyReginEgoLLCorr) -> [dyReginEgoLLCorr], CollisionRiskProbCalc/3(dyReginEgoLLCorr)
  EgoReginCorrCalc/2(dyReginEgoRUCorr) -> [dyReginEgoRUCorr], CollisionRiskProbCalc/2(dyReginEgoRUCorr)
  CollisionRiskProbCalc/1(dxPreCollison) -> Terminator1/1
  CollisionRiskProbCalc/2(dyPreCollison) -> Terminator2/1
  CollisionRiskProbCalc/3(collisionRiskProb) -> [collisionRiskProb], collisionRiskProb/1
  ObjAbsInfoCalc/1(velAbsObjCorr) -> ObjReginCorrCalc/1(velAbsObjCorr)
  tPredTime/1 -> Bus Creator/1  "tPredTime"
  ObjectLength/1 -> Bus Creator/3  "ObjectLength"
  ObjectWidth/1 -> Bus Creator/4  "ObjectWidth"
  ObjectYawAngle/1 -> Bus Creator/5  "ObjectYawAngle"
  vx/1 -> Bus Creator/6  "vx"
  vy/1 -> Bus Creator/7  "vy"
  Bus Creator/1 -> Bus Selector2/1, Bus Selector1/1, Bus Selector/1
  Bus Selector/1 -> EgoReginCalc/1(tPredTime)  "<tPredTime>"
  Bus Selector/2 -> EgoReginCalc/2(subjVel)  "<subjVel>"
  dx/1 -> Bus Creator/8  "dx"
  dy/1 -> Bus Creator/9  "dy"
  dxFrontBumper/1 -> Bus Creator/10  "dxFrontBumper"
  subjVel/1 -> Bus Creator/11  "subjVel"
  subjYawRate/1 -> Bus Creator/12  "subjYawRate"
  Bus Selector1/1 -> ObjAbsInfoCalc/1(vx)  "<vx>"
  Bus Selector1/2 -> ObjAbsInfoCalc/2(vy)  "<vy>"
  Bus Selector1/3 -> ObjAbsInfoCalc/3(dy)  "<dy>"
  Bus Selector1/4 -> ObjAbsInfoCalc/4(dxFrontBumper)  "<dxFrontBumper>"
  Bus Selector1/5 -> ObjAbsInfoCalc/5(subjYawRate)  "<subjYawRate>"
  Bus Selector1/6 -> ObjAbsInfoCalc/6(subjVel)  "<subjVel>"
  Bus Selector2/1 -> ObjReginCorrCalc/2(tPredTime)  "<tPredTime>"
  Bus Selector2/4 -> ObjReginCorrCalc/5(ObjectWidth)  "<ObjectWidth>"
  Bus Selector2/5 -> ObjReginCorrCalc/6(ObjectYawAngle)  "<ObjectYawAngle>"
  Bus Selector2/3 -> ObjReginCorrCalc/4(ObjectLength)  "<ObjectLength>"
  Bus Selector2/7 -> ObjReginCorrCalc/8(dx)  "<dx>"
  Bus Selector2/8 -> ObjReginCorrCalc/9(dy)  "<dy>"
  EgoReginCalc/1(dxReginEgoRU) -> EgoReginCorrCalc/1(dxReginEgoRU)
  EgoReginCalc/2(dyReginEgoRU) -> EgoReginCorrCalc/2(dyReginEgoRU)
  EgoReginCalc/3(dxReginEgoLL) -> EgoReginCorrCalc/3(dxReginEgoLL)
  EgoReginCalc/4(dyReginEgoLL) -> EgoReginCorrCalc/4(dyReginEgoLL)
  ObjReginCorrCalc/1(dxReginObjRUCorr) -> [dxReginObjRUCorr], CollisionRiskProbCalc/5(dxReginObjRUCorr)
  ObjReginCorrCalc/2(dyReginObjRUCorr) -> [dyReginObjRUCorr], CollisionRiskProbCalc/6(dyReginObjRUCorr)
  ObjReginCorrCalc/3(dxReginObjLLCorr) -> [dxReginObjLLCorr], CollisionRiskProbCalc/7(dxReginObjLLCorr)
  ObjReginCorrCalc/4(dyReginObjLLCorr) -> [dyReginObjLLCorr], CollisionRiskProbCalc/8(dyReginObjLLCorr)
  CollisionRiskProbCalc/4(dyCollisionRiskProb) -> [dyCollisionRiskProb], dyCollisionRiskProb/1
  CollisionRiskProbCalc/5(dxCollisionRiskProb) -> [dxCollisionRiskProb], dxCollisionRiskProb/1
  [dyReginObjRUCorr] -> MATLAB Function/6(dyReginObjRUCorr)
  [dxReginObjRUCorr] -> MATLAB Function/5(dxReginObjRUCorr)
  [dxReginObjLLCorr] -> MATLAB Function/7(dxReginObjLLCorr)
  [dyReginEgoLLCorr] -> MATLAB Function/3(dyReginEgoLLCorr)
  [dxReginEgoLLCorr] -> MATLAB Function/4(dxReginEgoLLCorr)
  [dxReginEgoRUCorr] -> MATLAB Function/1(dxReginEgoRUCorr)
  [dyReginEgoRUCorr] -> MATLAB Function/2(dyReginEgoRUCorr)
  [dyReginObjLLCorr] -> MATLAB Function/8(dyReginObjLLCorr)
  [dxCollisionRiskProb] -> MATLAB Function/11(dxCollisionRiskProb)
  [collisionRiskProb] -> MATLAB Function/9(collisionRiskProb)
  [dyCollisionRiskProb] -> MATLAB Function/10(dyCollisionRiskProb)
  ObjectMovingOrientation/1 -> Bus Creator/13  "ObjectMovingOrientation"
  Bus Selector2/6 -> ObjReginCorrCalc/7(ObjectMovingOrientation)  "<ObjectMovingOrientation>"
  ObjectTypeCameraConfirmed/1 -> Bus Creator/2  "ObjectTypeCameraConfirmed"
  Bus Selector2/2 -> ObjReginCorrCalc/3(ObjectTypeCameraConfirmed)  "<ObjectTypeCameraConfirmed>"

== system TosAeb/FcwAebJa_Step_sys/ALG_AEBSystem/ALG_AEBController/AEB_LatObjInfoCalcApp/LatPedObjCalc/LatPedObjCalc/latPedObjInfoCalc/ObjCrossFlgCal/PedPreCollisionCalcFunc ==
  tPredTime : Inport  OutDataTypeStr=single
  subjVel : Inport  Port=2
  subjLongAccel : Inport  Port=3
  subjLatAccel : Inport  Port=4
  subjYawRate : Inport  Port=5
  ObjectYawAngle : Inport  Port=6
  ObjectMovingOrientation : Inport  Port=7
  ObjectTypeCameraConfirmed : Inport  Port=8
  ObjectLength : Inport  Port=9
  ObjectWidth : Inport  Port=10
  dx : Inport  Port=11
  vy : Inport  Port=12
  ay : Inport  Port=13
  dy : Inport  Port=14
  vxAbsObj : Inport  Port=15
  axAbsObj : Inport  Port=16
  function : TriggerPort  TriggerType=function-call  VariantControl=Variant  PortDimensions=1
  ObjBoundingBox : Subsystem
  ObjLenthWidthCalc : Subsystem
  ObjPreReferPointCalc : Subsystem
  PreCrossFlgCalcFlow : Stateflow chart  TreatAsAtomicUnit=on
  PreRefPointEgoCalc : Subsystem
  SubjBoundingBox : Subsystem
  sincosCoefCalc : Subsystem
  sincosCoefCalc1 : Subsystem
  pedPreCollisionFlg : Outport
  wires:
  PreRefPointEgoCalc/3(YawAnglePre) -> sincosCoefCalc/1(angle)
  PreRefPointEgoCalc/2(" dyPreRefPoint") -> SubjBoundingBox/2(dyPreRefPoint)
  sincosCoefCalc/1(sincosCoef) -> SubjBoundingBox/3(sincosCoef)
  PreRefPointEgoCalc/1(" dxPreRefPoint") -> SubjBoundingBox/1(dxPreRefPoint)
  ObjPreReferPointCalc/3(YawAnglePreObj) -> sincosCoefCalc1/1(angle)
  ObjLenthWidthCalc/2(ObjWidthCorr) -> ObjBoundingBox/2(ObjWidthCorr)
  ObjLenthWidthCalc/1(ObjLengthCorr) -> ObjBoundingBox/1(ObjLengthCorr)
  ObjPreReferPointCalc/1(dxPreRefPointObj) -> ObjBoundingBox/3(dxPreRefPointObj)
  ObjPreReferPointCalc/2(dyPreRefPointObj) -> ObjBoundingBox/4(dyPreRefPointObj)
  sincosCoefCalc1/1(sincosCoef) -> ObjBoundingBox/5(sincosCoef)
  SubjBoundingBox/1(subjDxPre) -> PreCrossFlgCalcFlow/1(subjDxPre)
  SubjBoundingBox/2(subjDyPre) -> PreCrossFlgCalcFlow/2(subjDyPre)
  ObjBoundingBox/1(objDxPre) -> PreCrossFlgCalcFlow/3(objDxPre)
  ObjBoundingBox/2(objDyPre) -> PreCrossFlgCalcFlow/4(objDyPre)
  PreCrossFlgCalcFlow/1(pedPreCollisionFlg) -> pedPreCollisionFlg/1
  ObjectLength/1 -> ObjLenthWidthCalc/4(ObjectLength)
  ObjectWidth/1 -> ObjLenthWidthCalc/5(ObjectWidth)
  ObjectYawAngle/1 -> ObjPreReferPointCalc/9(ObjectYawAngle), ObjLenthWidthCalc/1(ObjectYawAngle)
  dx/1 -> ObjPreReferPointCalc/1(dx)
  vxAbsObj/1 -> ObjPreReferPointCalc/2(vxAbsObj)
  axAbsObj/1 -> ObjPreReferPointCalc/4(axAbsObj)
  vy/1 -> ObjPreReferPointCalc/5(vy)
  ay/1 -> ObjPreReferPointCalc/7(ay)
  dy/1 -> ObjPreReferPointCalc/8(dy)
  tPredTime/1 -> [tPredTime], PreRefPointEgoCalc/2(tPredTime)
  subjLongAccel/1 -> PreRefPointEgoCalc/3(subjLongAccel)
  subjVel/1 -> PreRefPointEgoCalc/1(subjVel)
  subjLatAccel/1 -> [subjLatAccel], PreRefPointEgoCalc/4(subjLatAccel)
  subjYawRate/1 -> PreRefPointEgoCalc/5(subjYawRate)
  [tPredTime] -> ObjPreReferPointCalc/3(tPredTime)
  [subjLatAccel] -> ObjPreReferPointCalc/6(subjLatAccel)
  ObjectMovingOrientation/1 -> ObjLenthWidthCalc/2(ObjectMovingOrientation)
  ObjectTypeCameraConfirmed/1 -> ObjLenthWidthCalc/3(ObjectTypeCameraConfirmed)
